simulink model "HvacMdlAirFlowDistbnFrnt"  (R2022b) (continued)
== system HvacMdlAirFlowDistbnFrnt == (continued)
  HvacAirMFlowEstimdForCtrlr_HvacAirMFlowEstimdForCtrlr/1 -> HvacMdlAirFlowDistbnFrnt_Runnable_sys/18(HvacAirMFlowEstimdForCtrlr_HvacAirMFlowEstimdForCtrlr_read)
  HvacCtrlrFanBoostReqLe_HvacCtrlrFanBoostReqLe/1 -> HvacMdlAirFlowDistbnFrnt_Runnable_sys/19(HvacCtrlrFanBoostReqLe_HvacCtrlrFanBoostReqLe_read)
  HvacCtrlrFanBoostReqRi_HvacCtrlrFanBoostReqRi/1 -> HvacMdlAirFlowDistbnFrnt_Runnable_sys/20(HvacCtrlrFanBoostReqRi_HvacCtrlrFanBoostReqRi_read)
  HvacCtrlrFanReqFrnt_HvacCtrlrFanReqFrnt/1 -> HvacMdlAirFlowDistbnFrnt_Runnable_sys/21(HvacCtrlrFanReqFrnt_HvacCtrlrFanReqFrnt_read)
  HvacCtrlrReqFrntForRowFirstForActrDefrst_HvacCtrlrReqFrntForRowFirstForActrDefrst/1 -> HvacMdlAirFlowDistbnFrnt_Runnable_sys/22(HvacCtrlrReqFrntForRowFirstForActrDefrst_HvacCtrlrReqFrntForRowFirstForActrDefrst_read)
  HvacCtrlrReqFrntForRowFirstForActrFlr_HvacCtrlrReqFrntForRowFirstForActrFlr/1 -> HvacMdlAirFlowDistbnFrnt_Runnable_sys/23(HvacCtrlrReqFrntForRowFirstForActrFlr_HvacCtrlrReqFrntForRowFirstForActrFlr_read)
  HvacCtrlrReqFrntForRowFirstForActrMod_HvacCtrlrReqFrntForRowFirstForActrMod/1 -> HvacMdlAirFlowDistbnFrnt_Runnable_sys/24(HvacCtrlrReqFrntForRowFirstForActrMod_HvacCtrlrReqFrntForRowFirstForActrMod_read)
  HvacCtrlrReqFrntForRowFirstForActrTLe_HvacCtrlrReqFrntForRowFirstForActrTLe/1 -> HvacMdlAirFlowDistbnFrnt_Runnable_sys/25(HvacCtrlrReqFrntForRowFirstForActrTLe_HvacCtrlrReqFrntForRowFirstForActrTLe_read)
  HvacCtrlrReqFrntForRowFirstForActrTRi_HvacCtrlrReqFrntForRowFirstForActrTRi/1 -> HvacMdlAirFlowDistbnFrnt_Runnable_sys/26(HvacCtrlrReqFrntForRowFirstForActrTRi_HvacCtrlrReqFrntForRowFirstForActrTRi_read)
  HvacCtrlrReqFrntForRowFirstForActrVent_HvacCtrlrReqFrntForRowFirstForActrVent/1 -> HvacMdlAirFlowDistbnFrnt_Runnable_sys/27(HvacCtrlrReqFrntForRowFirstForActrVent_HvacCtrlrReqFrntForRowFirstForActrVent_read)
  HvacCtrlrReqFrntForRowSecForActrFlr_HvacCtrlrReqFrntForRowSecForActrFlrLe/1 -> HvacMdlAirFlowDistbnFrnt_Runnable_sys/28(HvacCtrlrReqFrntForRowSecForActrFlr_HvacCtrlrReqFrntForRowSecForActrFlrLe_read)
  HvacCtrlrReqFrntForRowSecForActrTLe_HvacCtrlrReqFrntForRowSecForActrTLe/1 -> HvacMdlAirFlowDistbnFrnt_Runnable_sys/29(HvacCtrlrReqFrntForRowSecForActrTLe_HvacCtrlrReqFrntForRowSecForActrTLe_read)
  HvacCtrlrReqFrntForRowSecForActrTRi_HvacCtrlrReqFrntForRowSecForActrTRi/1 -> HvacMdlAirFlowDistbnFrnt_Runnable_sys/30(HvacCtrlrReqFrntForRowSecForActrTRi_HvacCtrlrReqFrntForRowSecForActrTRi_read)
  HvacCtrlrReqFrntForRowSecForActrVent_HvacCtrlrReqFrntForRowSecForActrVent/1 -> HvacMdlAirFlowDistbnFrnt_Runnable_sys/31(HvacCtrlrReqFrntForRowSecForActrVent_HvacCtrlrReqFrntForRowSecForActrVent_read)
  HvacFlowFrntReqForDefrst_HvacFlowFrntReqForDefrst/1 -> HvacMdlAirFlowDistbnFrnt_Runnable_sys/32(HvacFlowFrntReqForDefrst_HvacFlowFrntReqForDefrst_read)
  HvacFlowFrntReqForRowFirstAtFlr_HvacFlowFrntReqForRowFirstAtFlr/1 -> HvacMdlAirFlowDistbnFrnt_Runnable_sys/33(HvacFlowFrntReqForRowFirstAtFlr_HvacFlowFrntReqForRowFirstAtFlr_read)
  HvacFlowFrntReqForRowFirstAtFlr_HvacFlowFrntReqForRowFirstAtFlrLe/1 -> HvacMdlAirFlowDistbnFrnt_Runnable_sys/34(HvacFlowFrntReqForRowFirstAtFlr_HvacFlowFrntReqForRowFirstAtFlrLe_read)
  HvacFlowFrntReqForRowFirstAtFlr_HvacFlowFrntReqForRowFirstAtFlrRi/1 -> HvacMdlAirFlowDistbnFrnt_Runnable_sys/35(HvacFlowFrntReqForRowFirstAtFlr_HvacFlowFrntReqForRowFirstAtFlrRi_read)
  HvacFlowFrntReqForRowFirstAtVent_HvacFlowFrntReqForRowFirstAtVent/1 -> HvacMdlAirFlowDistbnFrnt_Runnable_sys/36(HvacFlowFrntReqForRowFirstAtVent_HvacFlowFrntReqForRowFirstAtVent_read)
  HvacFlowFrntReqForRowFirstAtVent_HvacFlowFrntReqForRowFirstAtVentLe/1 -> HvacMdlAirFlowDistbnFrnt_Runnable_sys/37(HvacFlowFrntReqForRowFirstAtVent_HvacFlowFrntReqForRowFirstAtVentLe_read)
  HvacFlowFrntReqForRowFirstAtVent_HvacFlowFrntReqForRowFirstAtVentLeMid/1 -> HvacMdlAirFlowDistbnFrnt_Runnable_sys/38(HvacFlowFrntReqForRowFirstAtVent_HvacFlowFrntReqForRowFirstAtVentLeMid_read)
  HvacFlowFrntReqForRowFirstAtVent_HvacFlowFrntReqForRowFirstAtVentLeSide/1 -> HvacMdlAirFlowDistbnFrnt_Runnable_sys/39(HvacFlowFrntReqForRowFirstAtVent_HvacFlowFrntReqForRowFirstAtVentLeSide_read)
  HvacFlowFrntReqForRowFirstAtVent_HvacFlowFrntReqForRowFirstAtVentRi/1 -> HvacMdlAirFlowDistbnFrnt_Runnable_sys/40(HvacFlowFrntReqForRowFirstAtVent_HvacFlowFrntReqForRowFirstAtVentRi_read)
  HvacFlowFrntReqForRowFirstAtVent_HvacFlowFrntReqForRowFirstAtVentRiMid/1 -> HvacMdlAirFlowDistbnFrnt_Runnable_sys/41(HvacFlowFrntReqForRowFirstAtVent_HvacFlowFrntReqForRowFirstAtVentRiMid_read)
  HvacFlowFrntReqForRowFirstAtVent_HvacFlowFrntReqForRowFirstAtVentRiSide/1 -> HvacMdlAirFlowDistbnFrnt_Runnable_sys/42(HvacFlowFrntReqForRowFirstAtVent_HvacFlowFrntReqForRowFirstAtVentRiSide_read)
  HvacFlowFrntReqForRowSecAtFlr_HvacFlowFrntReqForRowSecAtFlr/1 -> HvacMdlAirFlowDistbnFrnt_Runnable_sys/43(HvacFlowFrntReqForRowSecAtFlr_HvacFlowFrntReqForRowSecAtFlr_read)
  HvacFlowFrntReqForRowSecAtFlr_HvacFlowFrntReqForRowSecAtFlrLe/1 -> HvacMdlAirFlowDistbnFrnt_Runnable_sys/44(HvacFlowFrntReqForRowSecAtFlr_HvacFlowFrntReqForRowSecAtFlrLe_read)
  HvacFlowFrntReqForRowSecAtFlr_HvacFlowFrntReqForRowSecAtFlrRi/1 -> HvacMdlAirFlowDistbnFrnt_Runnable_sys/45(HvacFlowFrntReqForRowSecAtFlr_HvacFlowFrntReqForRowSecAtFlrRi_read)
  HvacFlowFrntReqForRowSecAtVent_HvacFlowFrntReqForRowSecAtVent/1 -> HvacMdlAirFlowDistbnFrnt_Runnable_sys/46(HvacFlowFrntReqForRowSecAtVent_HvacFlowFrntReqForRowSecAtVent_read)
  HvacFlowFrntReqForRowSecAtVent_HvacFlowFrntReqForRowSecAtVentLe/1 -> HvacMdlAirFlowDistbnFrnt_Runnable_sys/47(HvacFlowFrntReqForRowSecAtVent_HvacFlowFrntReqForRowSecAtVentLe_read)
  HvacFlowFrntReqForRowSecAtVent_HvacFlowFrntReqForRowSecAtVentRi/1 -> HvacMdlAirFlowDistbnFrnt_Runnable_sys/48(HvacFlowFrntReqForRowSecAtVent_HvacFlowFrntReqForRowSecAtVentRi_read)
  NotAllwdForMaxReqReqdForRowSecAtVent_NotAllwdForMaxReqReqdForRowSecAtVent/1 -> HvacMdlAirFlowDistbnFrnt_Runnable_sys/49(NotAllwdForMaxReqReqdForRowSecAtVent_NotAllwdForMaxReqReqdForRowSecAtVent_read)
  OvrdForMaxReqForActrForDefrst_OvrdForMaxReqForActrForDefrst/1 -> HvacMdlAirFlowDistbnFrnt_Runnable_sys/50(OvrdForMaxReqForActrForDefrst_OvrdForMaxReqForActrForDefrst_read)
  OvrdForMaxReqForActrForRowFirstAtFlr_OvrdForMaxReqForActrForRowFirstAtFlr/1 -> HvacMdlAirFlowDistbnFrnt_Runnable_sys/51(OvrdForMaxReqForActrForRowFirstAtFlr_OvrdForMaxReqForActrForRowFirstAtFlr_read)
  OvrdForMaxReqForActrForRowFirstAtFlr_OvrdForMaxReqForActrForRowFirstAtFlrLe/1 -> HvacMdlAirFlowDistbnFrnt_Runnable_sys/52(OvrdForMaxReqForActrForRowFirstAtFlr_OvrdForMaxReqForActrForRowFirstAtFlrLe_read)
  OvrdForMaxReqForActrForRowFirstAtFlr_OvrdForMaxReqForActrForRowFirstAtFlrRi/1 -> HvacMdlAirFlowDistbnFrnt_Runnable_sys/53(OvrdForMaxReqForActrForRowFirstAtFlr_OvrdForMaxReqForActrForRowFirstAtFlrRi_read)
  OvrdForMaxReqForActrForRowFirstAtVent_OvrdForMaxReqForActrForRowFirstAtVent/1 -> HvacMdlAirFlowDistbnFrnt_Runnable_sys/54(OvrdForMaxReqForActrForRowFirstAtVent_OvrdForMaxReqForActrForRowFirstAtVent_read)
  OvrdForMaxReqForActrForRowFirstAtVent_OvrdForMaxReqForActrForRowFirstAtVentLe/1 -> HvacMdlAirFlowDistbnFrnt_Runnable_sys/55(OvrdForMaxReqForActrForRowFirstAtVent_OvrdForMaxReqForActrForRowFirstAtVentLe_read)
  OvrdForMaxReqForActrForRowFirstAtVent_OvrdForMaxReqForActrForRowFirstAtVentRi/1 -> HvacMdlAirFlowDistbnFrnt_Runnable_sys/56(OvrdForMaxReqForActrForRowFirstAtVent_OvrdForMaxReqForActrForRowFirstAtVentRi_read)
  OvrdForMaxReqForActrForRowFirstAtVent_OvrdForMaxReqForActrForRowFirstAtVentSideLe/1 -> HvacMdlAirFlowDistbnFrnt_Runnable_sys/57(OvrdForMaxReqForActrForRowFirstAtVent_OvrdForMaxReqForActrForRowFirstAtVentSideLe_read)
  OvrdForMaxReqForActrForRowFirstAtVent_OvrdForMaxReqForActrForRowFirstAtVentSideRi/1 -> HvacMdlAirFlowDistbnFrnt_Runnable_sys/58(OvrdForMaxReqForActrForRowFirstAtVent_OvrdForMaxReqForActrForRowFirstAtVentSideRi_read)
  OvrdForMaxReqForActrForRowSecAtFlr_OvrdForMaxReqForActrForRowSecAtFlr/1 -> HvacMdlAirFlowDistbnFrnt_Runnable_sys/59(OvrdForMaxReqForActrForRowSecAtFlr_OvrdForMaxReqForActrForRowSecAtFlr_read)
  OvrdForMaxReqForActrForRowSecAtFlr_OvrdForMaxReqForActrForRowSecAtFlrLe/1 -> HvacMdlAirFlowDistbnFrnt_Runnable_sys/60(OvrdForMaxReqForActrForRowSecAtFlr_OvrdForMaxReqForActrForRowSecAtFlrLe_read)
  OvrdForMaxReqForActrForRowSecAtFlr_OvrdForMaxReqForActrForRowSecAtFlrRi/1 -> HvacMdlAirFlowDistbnFrnt_Runnable_sys/61(OvrdForMaxReqForActrForRowSecAtFlr_OvrdForMaxReqForActrForRowSecAtFlrRi_read)
  OvrdForMaxReqForActrForRowSecAtVent_OvrdForMaxReqForActrForRowSecAtVent/1 -> HvacMdlAirFlowDistbnFrnt_Runnable_sys/62(OvrdForMaxReqForActrForRowSecAtVent_OvrdForMaxReqForActrForRowSecAtVent_read)
  OvrdForMaxReqForActrForRowSecAtVent_OvrdForMaxReqForActrForRowSecAtVentLe/1 -> HvacMdlAirFlowDistbnFrnt_Runnable_sys/63(OvrdForMaxReqForActrForRowSecAtVent_OvrdForMaxReqForActrForRowSecAtVentLe_read)
  OvrdForMaxReqForActrForRowSecAtVent_OvrdForMaxReqForActrForRowSecAtVentRi/1 -> HvacMdlAirFlowDistbnFrnt_Runnable_sys/64(OvrdForMaxReqForActrForRowSecAtVent_OvrdForMaxReqForActrForRowSecAtVentRi_read)
  ClimaCfgForNrOfTSetg1_ClimaCfgForNrOfTSetg1/1 -> HvacMdlAirFlowDistbnFrnt_Runnable_sys/65(ClimaCfgForNrOfTSetg1_ClimaCfgForNrOfTSetg1_read)
  HvacCtrlrReqFrntForRowFirstForActrFlr_HvacCtrlrReqFrntForRowFirstForActrFlrLe/1 -> HvacMdlAirFlowDistbnFrnt_Runnable_sys/66(HvacCtrlrReqFrntForRowFirstForActrFlr_HvacCtrlrReqFrntForRowFirstForActrFlrLe_read)
  HvacCtrlrReqFrntForRowFirstForActrFlr_HvacCtrlrReqFrntForRowFirstForActrFlrRi/1 -> HvacMdlAirFlowDistbnFrnt_Runnable_sys/67(HvacCtrlrReqFrntForRowFirstForActrFlr_HvacCtrlrReqFrntForRowFirstForActrFlrRi_read)
  HvacCtrlrReqFrntForRowFirstForActrVent_HvacCtrlrReqFrntForRowFirstForActrVentLe/1 -> HvacMdlAirFlowDistbnFrnt_Runnable_sys/68(HvacCtrlrReqFrntForRowFirstForActrVent_HvacCtrlrReqFrntForRowFirstForActrVentLe_read)
  HvacCtrlrReqFrntForRowFirstForActrVent_HvacCtrlrReqFrntForRowFirstForActrVentRi/1 -> HvacMdlAirFlowDistbnFrnt_Runnable_sys/69(HvacCtrlrReqFrntForRowFirstForActrVent_HvacCtrlrReqFrntForRowFirstForActrVentRi_read)
  HvacCtrlrReqFrntForRowFirstForActrVent_HvacCtrlrReqFrntForRowFirstForActrVentSideLe/1 -> HvacMdlAirFlowDistbnFrnt_Runnable_sys/70(HvacCtrlrReqFrntForRowFirstForActrVent_HvacCtrlrReqFrntForRowFirstForActrVentSideLe_read)
  HvacCtrlrReqFrntForRowFirstForActrVent_HvacCtrlrReqFrntForRowFirstForActrVentSideRi/1 -> HvacMdlAirFlowDistbnFrnt_Runnable_sys/71(HvacCtrlrReqFrntForRowFirstForActrVent_HvacCtrlrReqFrntForRowFirstForActrVentSideRi_read)
  HvacCtrlrReqFrntForRowFirstLeForActrMod_HvacCtrlrReqFrntForRowFirstLeForActrMod/1 -> HvacMdlAirFlowDistbnFrnt_Runnable_sys/72(HvacCtrlrReqFrntForRowFirstLeForActrMod_HvacCtrlrReqFrntForRowFirstLeForActrMod_read)
  HvacCtrlrReqFrntForRowSecForActrFlr_HvacCtrlrReqFrntForRowSecForActrFlr/1 -> HvacMdlAirFlowDistbnFrnt_Runnable_sys/73(HvacCtrlrReqFrntForRowSecForActrFlr_HvacCtrlrReqFrntForRowSecForActrFlr_read)
  HvacCtrlrReqFrntForRowSecForActrFlr_HvacCtrlrReqFrntForRowSecForActrFlrRi/1 -> HvacMdlAirFlowDistbnFrnt_Runnable_sys/74(HvacCtrlrReqFrntForRowSecForActrFlr_HvacCtrlrReqFrntForRowSecForActrFlrRi_read)
  HvacCtrlrReqFrntForRowSecForActrModLe_HvacCtrlrReqFrntForRowSecForActrModLe/1 -> HvacMdlAirFlowDistbnFrnt_Runnable_sys/75(HvacCtrlrReqFrntForRowSecForActrModLe_HvacCtrlrReqFrntForRowSecForActrModLe_read)
  HvacCtrlrReqFrntForRowSecForActrModRi_HvacCtrlrReqFrntForRowSecForActrModRi/1 -> HvacMdlAirFlowDistbnFrnt_Runnable_sys/76(HvacCtrlrReqFrntForRowSecForActrModRi_HvacCtrlrReqFrntForRowSecForActrModRi_read)
  ExtVentToastFrmClima_Prkg_ExtVentToastFrmClima/1 -> HvacMdlAirFlowDistbnFrnt_Runnable_sys/77(ExtVentToastFrmClima_Prkg_ExtVentToastFrmClima_read)
  ActrPosnFrntActForRowFirstModDistbnLe_ActrPosnFrntActForRowFirstModDistbnLe/1 -> HvacMdlAirFlowDistbnFrnt_Runnable_sys/78(ActrPosnFrntActForRowFirstModDistbnLe_ActrPosnFrntActForRowFirstModDistbnLe_read)
  ActrPosnFrntActForRowSecModDistbnLe_ActrPosnFrntActForRowSecModDistbnLe/1 -> HvacMdlAirFlowDistbnFrnt_Runnable_sys/79(ActrPosnFrntActForRowSecModDistbnLe_ActrPosnFrntActForRowSecModDistbnLe_read)
  ActrPosnFrntActForRowSecModDistbnRi_ActrPosnFrntActForRowSecModDistbnRi/1 -> HvacMdlAirFlowDistbnFrnt_Runnable_sys/80(ActrPosnFrntActForRowSecModDistbnRi_ActrPosnFrntActForRowSecModDistbnRi_read)
  AirFlapPosnFrntActForRowFirstForActrDefrst_AirFlapPosnFrntActForRowFirstForActrDefrst/1 -> HvacMdlAirFlowDistbnFrnt_Runnable_sys/81(AirFlapPosnFrntActForRowFirstForActrDefrst_AirFlapPosnFrntActForRowFirstForActrDefrst_read)
  ClimaAtRowSecActvNgcc_ClimaAtRowSecActv/1 -> HvacMdlAirFlowDistbnFrnt_Runnable_sys/82(ClimaAtRowSecActvNgcc_ClimaAtRowSecActv_read)
  ClimaAtRowSecActvNgcc_ClimaAtRowSecActvLe/1 -> HvacMdlAirFlowDistbnFrnt_Runnable_sys/83(ClimaAtRowSecActvNgcc_ClimaAtRowSecActvLe_read)
  ClimaAtRowSecActvNgcc_ClimaAtRowSecActvRi/1 -> HvacMdlAirFlowDistbnFrnt_Runnable_sys/84(ClimaAtRowSecActvNgcc_ClimaAtRowSecActvRi_read)
  ClimaCfgForHvacRePrsnt1_ClimaCfgForHvacRePrsnt1/1 -> HvacMdlAirFlowDistbnFrnt_Runnable_sys/85(ClimaCfgForHvacRePrsnt1_ClimaCfgForHvacRePrsnt1_read)
  ClimaCfgForPTCInFrontHVAC_ClimaCfgForPTCInFrontHVAC/1 -> HvacMdlAirFlowDistbnFrnt_Runnable_sys/86(ClimaCfgForPTCInFrontHVAC_ClimaCfgForPTCInFrontHVAC_read)
  ClimaCfgHvacForFrntLeTempActr_ClimaCfgHvacForFrntLeTempActr/1 -> HvacMdlAirFlowDistbnFrnt_Runnable_sys/87(ClimaCfgHvacForFrntLeTempActr_ClimaCfgHvacForFrntLeTempActr_read)
  ClimaCfgHvacForFrntRiTempActr_ClimaCfgHvacForFrntRiTempActr/1 -> HvacMdlAirFlowDistbnFrnt_Runnable_sys/88(ClimaCfgHvacForFrntRiTempActr_ClimaCfgHvacForFrntRiTempActr_read)
  ClimaCfgReqVersionReplace_ClimaCfgReqVersionReplace/1 -> HvacMdlAirFlowDistbnFrnt_Runnable_sys/89(ClimaCfgReqVersionReplace_ClimaCfgReqVersionReplace_read)
  HvacTempFlapActlPosnFrstRowLe_HvacTempFlapActlPosnFrstRowLe/1 -> HvacMdlAirFlowDistbnFrnt_Runnable_sys/90(HvacTempFlapActlPosnFrstRowLe_HvacTempFlapActlPosnFrstRowLe_read)
  HvacTempFlapActlPosnFrstRowRi_HvacTempFlapActlPosnFrstRowRi/1 -> HvacMdlAirFlowDistbnFrnt_Runnable_sys/91(HvacTempFlapActlPosnFrstRowRi_HvacTempFlapActlPosnFrstRowRi_read)
  HvacTempFlapActlPosnSecRowLe_HvacTempFlapActlPosnSecRowLe/1 -> HvacMdlAirFlowDistbnFrnt_Runnable_sys/92(HvacTempFlapActlPosnSecRowLe_HvacTempFlapActlPosnSecRowLe_read)
  HvacTempFlapActlPosnSecRowRi_HvacTempFlapActlPosnSecRowRi/1 -> HvacMdlAirFlowDistbnFrnt_Runnable_sys/93(HvacTempFlapActlPosnSecRowRi_HvacTempFlapActlPosnSecRowRi_read)
  ManModeForRowFirstLeForModelDial_ManModeForRowFirstLeForModelDial/1 -> HvacMdlAirFlowDistbnFrnt_Runnable_sys/94(ManModeForRowFirstLeForModelDial_ManModeForRowFirstLeForModelDial_read)
  ManModeForRowFirstRiForModelDial_ManModeForRowFirstRiForModelDial/1 -> HvacMdlAirFlowDistbnFrnt_Runnable_sys/95(ManModeForRowFirstRiForModelDial_ManModeForRowFirstRiForModelDial_read)
  ManModeForRowSecLeForModelDial_ManModeForRowSecLeForModelDial/1 -> HvacMdlAirFlowDistbnFrnt_Runnable_sys/96(ManModeForRowSecLeForModelDial_ManModeForRowSecLeForModelDial_read)
  ManModeForRowSecRiForModelDial_ManModeForRowSecRiForModelDial/1 -> HvacMdlAirFlowDistbnFrnt_Runnable_sys/97(ManModeForRowSecRiForModelDial_ManModeForRowSecRiForModelDial_read)
  HvacMdlAirFlowDistbnFrnt_Runnable_sys/1(HvacHeatElmAirMFlowEstimdFirstLeNgcc_HvacHeatElmAirMFlowEstimdFirstLeNgcc_write) -> HvacHeatElmAirMFlowEstimdFirstLeNgcc_HvacHeatElmAirMFlowEstimdFirstLeNgcc/1
  HvacMdlAirFlowDistbnFrnt_Runnable_sys/2(HvacHeatElmAirMFlowEstimdFirstRiNgcc_HvacHeatElmAirMFlowEstimdFirstRiNgcc_write) -> HvacHeatElmAirMFlowEstimdFirstRiNgcc_HvacHeatElmAirMFlowEstimdFirstRiNgcc/1
  HvacMdlAirFlowDistbnFrnt_Runnable_sys/3(HvacHeatElmAirMFlowEstimdSecLeNgcc_HvacHeatElmAirMFlowEstimdSecLeNgcc_write) -> HvacHeatElmAirMFlowEstimdSecLeNgcc_HvacHeatElmAirMFlowEstimdSecLeNgcc/1
  HvacMdlAirFlowDistbnFrnt_Runnable_sys/4(FacOfSecVent_FacOfSecVent_write) -> FacOfSecVent_FacOfSecVent/1
  HvacMdlAirFlowDistbnFrnt_Runnable_sys/5(FacOfVentLeFlow_FacOfVentLeFlow_write) -> FacOfVentLeFlow_FacOfVentLeFlow/1
  HvacMdlAirFlowDistbnFrnt_Runnable_sys/6(FacOfVentRiFlow_FacOfVentRiFlow_write) -> FacOfVentRiFlow_FacOfVentRiFlow/1
  HvacMdlAirFlowDistbnFrnt_Runnable_sys/7(FacOfVentSideLeFlow_FacOfVentSideLeFlow_write) -> FacOfVentSideLeFlow_FacOfVentSideLeFlow/1
  HvacMdlAirFlowDistbnFrnt_Runnable_sys/8(FacOfVentSideRiFlow_FacOfVentSideRiFlow_write) -> FacOfVentSideRiFlow_FacOfVentSideRiFlow/1
  HvacMdlAirFlowDistbnFrnt_Runnable_sys/9(HvacAirFlowAddlForFanBoost_HvacAirFlowAddlForFanBoost_write) -> HvacAirFlowAddlForFanBoost_HvacAirFlowAddlForFanBoost/1
  HvacMdlAirFlowDistbnFrnt_Runnable_sys/10(HvacAirMFlowEstimdInHeatElm_HvacAirMFlowEstimdInHeatElm_write) -> HvacAirMFlowEstimdInHeatElm_HvacAirMFlowEstimdInHeatElm/1
  HvacMdlAirFlowDistbnFrnt_Runnable_sys/11(HvacFlowFrntActForDefrst_HvacFlowFrntActForDefrst_write) -> HvacFlowFrntActForDefrst_HvacFlowFrntActForDefrst/1
  HvacMdlAirFlowDistbnFrnt_Runnable_sys/12(HvacFlowFrntActForRowFirstAtFlr_HvacFlowFrntActForRowFirstAtFlr_write) -> HvacFlowFrntActForRowFirstAtFlr_HvacFlowFrntActForRowFirstAtFlr/1
  HvacMdlAirFlowDistbnFrnt_Runnable_sys/13(HvacFlowFrntActForRowFirstAtVent_HvacFlowFrntActForRowFirstAtVent_write) -> HvacFlowFrntActForRowFirstAtVent_HvacFlowFrntActForRowFirstAtVent/1
  HvacMdlAirFlowDistbnFrnt_Runnable_sys/14(HvacFlowFrntActForRowSecAtFlr_HvacFlowFrntActForRowSecAtFlr_write) -> HvacFlowFrntActForRowSecAtFlr_HvacFlowFrntActForRowSecAtFlr/1
  HvacMdlAirFlowDistbnFrnt_Runnable_sys/15(HvacFlowFrntActForRowSecAtVent_HvacFlowFrntActForRowSecAtVent_write) -> HvacFlowFrntActForRowSecAtVent_HvacFlowFrntActForRowSecAtVent/1
  HvacMdlAirFlowDistbnFrnt_Runnable_sys/16(HvacFlowFrntActForRowSecAtVent_HvacFlowFrntActForRowSecAtVentLe_write) -> HvacFlowFrntActForRowSecAtVent_HvacFlowFrntActForRowSecAtVentLe/1
  HvacMdlAirFlowDistbnFrnt_Runnable_sys/17(HvacFlowFrntActForRowSecAtVent_HvacFlowFrntActForRowSecAtVentRi_write) -> HvacFlowFrntActForRowSecAtVent_HvacFlowFrntActForRowSecAtVentRi/1
  HvacMdlAirFlowDistbnFrnt_Runnable_sys/18(HvacFlowFrntActForRowThrdAtFlr_HvacFlowFrntActForRowThrdAtFlr_write) -> HvacFlowFrntActForRowThrdAtFlr_HvacFlowFrntActForRowThrdAtFlr/1
  HvacMdlAirFlowDistbnFrnt_Runnable_sys/19(HvacFlowFrntForRowFirstAtDefrstLe_HvacFlowFrntForRowFirstAtDefrstLe_write) -> HvacFlowFrntForRowFirstAtDefrstLe_HvacFlowFrntForRowFirstAtDefrstLe/1
  HvacMdlAirFlowDistbnFrnt_Runnable_sys/20(HvacFlowFrntForRowFirstAtDefrstRi_HvacFlowFrntForRowFirstAtDefrstRi_write) -> HvacFlowFrntForRowFirstAtDefrstRi_HvacFlowFrntForRowFirstAtDefrstRi/1
  HvacMdlAirFlowDistbnFrnt_Runnable_sys/21(HvacFlowFrntForRowFirstAtDefrstSideLe_HvacFlowFrntForRowFirstAtDefrstSideLe_write) -> HvacFlowFrntForRowFirstAtDefrstSideLe_HvacFlowFrntForRowFirstAtDefrstSideLe/1
  HvacMdlAirFlowDistbnFrnt_Runnable_sys/22(HvacFlowFrntForRowFirstAtDefrstSideRi_HvacFlowFrntForRowFirstAtDefrstSideRi_write) -> HvacFlowFrntForRowFirstAtDefrstSideRi_HvacFlowFrntForRowFirstAtDefrstSideRi/1
  HvacMdlAirFlowDistbnFrnt_Runnable_sys/23(HvacFlowFrntForRowFirstAtFlrLe_HvacFlowFrntForRowFirstAtFlrLe_write) -> HvacFlowFrntForRowFirstAtFlrLe_HvacFlowFrntForRowFirstAtFlrLe/1
  HvacMdlAirFlowDistbnFrnt_Runnable_sys/24(HvacFlowFrntForRowFirstAtFlrRi_HvacFlowFrntForRowFirstAtFlrRi_write) -> HvacFlowFrntForRowFirstAtFlrRi_HvacFlowFrntForRowFirstAtFlrRi/1
  HvacMdlAirFlowDistbnFrnt_Runnable_sys/25(HvacFlowFrntForRowFirstAtVentLe_HvacFlowFrntForRowFirstAtVentLe_write) -> HvacFlowFrntForRowFirstAtVentLe_HvacFlowFrntForRowFirstAtVentLe/1
  HvacMdlAirFlowDistbnFrnt_Runnable_sys/26(HvacFlowFrntForRowFirstAtVentRi_HvacFlowFrntForRowFirstAtVentRi_write) -> HvacFlowFrntForRowFirstAtVentRi_HvacFlowFrntForRowFirstAtVentRi/1
  HvacMdlAirFlowDistbnFrnt_Runnable_sys/27(HvacFlowFrntForRowFirstAtVentSideLe_HvacFlowFrntForRowFirstAtVentSideLe_write) -> HvacFlowFrntForRowFirstAtVentSideLe_HvacFlowFrntForRowFirstAtVentSideLe/1
  HvacMdlAirFlowDistbnFrnt_Runnable_sys/28(HvacFlowFrntForRowFirstAtVentSideRi_HvacFlowFrntForRowFirstAtVentSideRi_write) -> HvacFlowFrntForRowFirstAtVentSideRi_HvacFlowFrntForRowFirstAtVentSideRi/1
  HvacMdlAirFlowDistbnFrnt_Runnable_sys/29(HvacFlowFrntForRowSecAtFlrLe_HvacFlowFrntForRowSecAtFlrLe_write) -> HvacFlowFrntForRowSecAtFlrLe_HvacFlowFrntForRowSecAtFlrLe/1
  HvacMdlAirFlowDistbnFrnt_Runnable_sys/30(HvacFlowFrntForRowSecAtFlrRi_HvacFlowFrntForRowSecAtFlrRi_write) -> HvacFlowFrntForRowSecAtFlrRi_HvacFlowFrntForRowSecAtFlrRi/1
  HvacMdlAirFlowDistbnFrnt_Runnable_sys/31(HvacFlowFrntForRowSecAtVentLe_HvacFlowFrntForRowSecAtVentLe_write) -> HvacFlowFrntForRowSecAtVentLe_HvacFlowFrntForRowSecAtVentLe/1
  HvacMdlAirFlowDistbnFrnt_Runnable_sys/32(HvacFlowFrntForRowSecAtVentRi_HvacFlowFrntForRowSecAtVentRi_write) -> HvacFlowFrntForRowSecAtVentRi_HvacFlowFrntForRowSecAtVentRi/1
  HvacMdlAirFlowDistbnFrnt_Runnable_sys/33(HvacFlowFrntForRowSecAtVentSideLe_HvacFlowFrntForRowSecAtVentSideLe_write) -> HvacFlowFrntForRowSecAtVentSideLe_HvacFlowFrntForRowSecAtVentSideLe/1
  HvacMdlAirFlowDistbnFrnt_Runnable_sys/34(HvacFlowFrntForRowSecAtVentSideRi_HvacFlowFrntForRowSecAtVentSideRi_write) -> HvacFlowFrntForRowSecAtVentSideRi_HvacFlowFrntForRowSecAtVentSideRi/1
  HvacMdlAirFlowDistbnFrnt_Runnable_sys/35(HvacFlowFrntForRowThrdAtFlrLe_HvacFlowFrntForRowThrdAtFlrLe_write) -> HvacFlowFrntForRowThrdAtFlrLe_HvacFlowFrntForRowThrdAtFlrLe/1
  HvacMdlAirFlowDistbnFrnt_Runnable_sys/36(HvacFlowFrntForRowThrdAtFlrRi_HvacFlowFrntForRowThrdAtFlrRi_write) -> HvacFlowFrntForRowThrdAtFlrRi_HvacFlowFrntForRowThrdAtFlrRi/1
  HvacMdlAirFlowDistbnFrnt_Runnable_sys/37(HvacFlrsFrnt_HvacFlrsFrnt_write) -> HvacFlrsFrnt_HvacFlrsFrnt/1
  HvacMdlAirFlowDistbnFrnt_Runnable_sys/38(MaxReqReqdFrntForDefrst_MaxReqReqdFrntForDefrst_write) -> MaxReqReqdFrntForDefrst_MaxReqReqdFrntForDefrst/1
  HvacMdlAirFlowDistbnFrnt_Runnable_sys/39(MaxReqReqdFrntForRowFirstAtFlr_MaxReqReqdFrntForRowFirstAtFlr_write) -> MaxReqReqdFrntForRowFirstAtFlr_MaxReqReqdFrntForRowFirstAtFlr/1
  HvacMdlAirFlowDistbnFrnt_Runnable_sys/40(MaxReqReqdFrntForRowFirstAtFlr_MaxReqReqdFrntForRowFirstAtFlrLe_write) -> MaxReqReqdFrntForRowFirstAtFlr_MaxReqReqdFrntForRowFirstAtFlrLe/1
  HvacMdlAirFlowDistbnFrnt_Runnable_sys/41(MaxReqReqdFrntForRowFirstAtFlr_MaxReqReqdFrntForRowFirstAtFlrRi_write) -> MaxReqReqdFrntForRowFirstAtFlr_MaxReqReqdFrntForRowFirstAtFlrRi/1
  HvacMdlAirFlowDistbnFrnt_Runnable_sys/42(MaxReqReqdFrntForRowFirstAtVent_MaxReqReqdFrntForRowFirstAtVent_write) -> MaxReqReqdFrntForRowFirstAtVent_MaxReqReqdFrntForRowFirstAtVent/1
  HvacMdlAirFlowDistbnFrnt_Runnable_sys/43(MaxReqReqdFrntForRowSecAtFlr_MaxReqReqdFrntForRowSecAtFlr_write) -> MaxReqReqdFrntForRowSecAtFlr_MaxReqReqdFrntForRowSecAtFlr/1
  HvacMdlAirFlowDistbnFrnt_Runnable_sys/44(MaxReqReqdFrntForRowSecAtVent_MaxReqReqdFrntForRowSecAtVent_write) -> MaxReqReqdFrntForRowSecAtVent_MaxReqReqdFrntForRowSecAtVent/1
  HvacMdlAirFlowDistbnFrnt_Runnable_sys/45(VCCMaxReqReqdForActrFrntEnumReserved_VCCMaxReqReqdForActrFrntEnumReserved_write) -> VCCMaxReqReqdForActrFrntEnumReserved_VCCMaxReqReqdForActrFrntEnumReserved/1
  HvacMdlAirFlowDistbnFrnt_Runnable_sys/46(HvacFlowFrntActForRowFirstAtFlr_HvacFlowFrntActForRowFirstAtFlrLe_write) -> HvacFlowFrntActForRowFirstAtFlr_HvacFlowFrntActForRowFirstAtFlrLe/1
  HvacMdlAirFlowDistbnFrnt_Runnable_sys/47(HvacFlowFrntActForRowFirstAtFlr_HvacFlowFrntActForRowFirstAtFlrRi_write) -> HvacFlowFrntActForRowFirstAtFlr_HvacFlowFrntActForRowFirstAtFlrRi/1
  HvacMdlAirFlowDistbnFrnt_Runnable_sys/48(HvacFlowFrntActForRowFirstAtVent_HvacFlowFrntActForRowFirstAtVentLe_write) -> HvacFlowFrntActForRowFirstAtVent_HvacFlowFrntActForRowFirstAtVentLe/1
  HvacMdlAirFlowDistbnFrnt_Runnable_sys/49(HvacFlowFrntActForRowFirstAtVent_HvacFlowFrntActForRowFirstAtVentRi_write) -> HvacFlowFrntActForRowFirstAtVent_HvacFlowFrntActForRowFirstAtVentRi/1
  HvacMdlAirFlowDistbnFrnt_Runnable_sys/50(HvacFlowFrntActForRowFirstAtVent_HvacFlowFrntActForRowFirstAtVentSideLe_write) -> HvacFlowFrntActForRowFirstAtVent_HvacFlowFrntActForRowFirstAtVentSideLe/1
  HvacMdlAirFlowDistbnFrnt_Runnable_sys/51(HvacFlowFrntActForRowFirstAtVent_HvacFlowFrntActForRowFirstAtVentSideRi_write) -> HvacFlowFrntActForRowFirstAtVent_HvacFlowFrntActForRowFirstAtVentSideRi/1
  HvacMdlAirFlowDistbnFrnt_Runnable_sys/52(HvacFlowFrntActForRowSecAtFlr_HvacFlowFrntActForRowSecAtFlrLe_write) -> HvacFlowFrntActForRowSecAtFlr_HvacFlowFrntActForRowSecAtFlrLe/1
  HvacMdlAirFlowDistbnFrnt_Runnable_sys/53(HvacFlowFrntActForRowSecAtFlr_HvacFlowFrntActForRowSecAtFlrRi_write) -> HvacFlowFrntActForRowSecAtFlr_HvacFlowFrntActForRowSecAtFlrRi/1
  MaxReqReqdFrntForRowFirstAtVent_MaxReqReqdFrntForRowFirstAtVentLe_merge/1 -> MaxReqReqdFrntForRowFirstAtVent_MaxReqReqdFrntForRowFirstAtVentLe/1
  MaxReqReqdFrntForRowFirstAtVent_MaxReqReqdFrntForRowFirstAtVentRi_merge/1 -> MaxReqReqdFrntForRowFirstAtVent_MaxReqReqdFrntForRowFirstAtVentRi/1
  HvacMdlAirFlowDistbnFrnt_Runnable_sys/56(MaxReqReqdFrntForRowFirstAtVent_MaxReqReqdFrntForRowFirstAtVentSideLe_write) -> MaxReqReqdFrntForRowFirstAtVent_MaxReqReqdFrntForRowFirstAtVentSideLe/1
  HvacMdlAirFlowDistbnFrnt_Runnable_sys/57(MaxReqReqdFrntForRowFirstAtVent_MaxReqReqdFrntForRowFirstAtVentSideRi_write) -> MaxReqReqdFrntForRowFirstAtVent_MaxReqReqdFrntForRowFirstAtVentSideRi/1
  HvacMdlAirFlowDistbnFrnt_Runnable_sys/58(MaxReqReqdFrntForRowSecAtFlr_MaxReqReqdFrntForRowSecAtFlrLe_write) -> MaxReqReqdFrntForRowSecAtFlr_MaxReqReqdFrntForRowSecAtFlrLe/1
  HvacMdlAirFlowDistbnFrnt_Runnable_sys/59(MaxReqReqdFrntForRowSecAtFlr_MaxReqReqdFrntForRowSecAtFlrRi_write) -> MaxReqReqdFrntForRowSecAtFlr_MaxReqReqdFrntForRowSecAtFlrRi/1
  HvacMdlAirFlowDistbnFrnt_Runnable_sys/60(MaxReqReqdFrntForRowSecAtVent_MaxReqReqdFrntForRowSecAtVentLe_write) -> MaxReqReqdFrntForRowSecAtVent_MaxReqReqdFrntForRowSecAtVentLe/1
  HvacMdlAirFlowDistbnFrnt_Runnable_sys/61(MaxReqReqdFrntForRowSecAtVent_MaxReqReqdFrntForRowSecAtVentRi_write) -> MaxReqReqdFrntForRowSecAtVent_MaxReqReqdFrntForRowSecAtVentRi/1
  HvacMdlAirFlowDistbnFrnt_Runnable_sys/55(MaxReqReqdFrntForRowFirstAtVent_MaxReqReqdFrntForRowFirstAtVentRi_write) -> [MaxReqReqdFrntForRowFirstAtVent_MaxReqReqdFrnt_a0f2095ec0cb5985]
  [MaxReqReqdFrntForRowFirstAtVent_MaxReqReqdFrnt_a0f2095ec0cb5985] -> MaxReqReqdFrntForRowFirstAtVent_MaxReqReqdFrntForRowFirstAtVentRi_merge/2
  HvacMdlAirFlowDistbnFrnt_Init/2(MaxReqReqdFrntForRowFirstAtVent_MaxReqReqdFrntForRowFirstAtVentRi_write) -> [MaxReqReqdFrntForRowFirstAtVent_MaxReqReqdFrnt_1a730eb18aa1a969]
  [MaxReqReqdFrntForRowFirstAtVent_MaxReqReqdFrnt_1a730eb18aa1a969] -> MaxReqReqdFrntForRowFirstAtVent_MaxReqReqdFrntForRowFirstAtVentRi_merge/1
  HvacMdlAirFlowDistbnFrnt_Runnable_sys/54(MaxReqReqdFrntForRowFirstAtVent_MaxReqReqdFrntForRowFirstAtVentLe_write) -> [MaxReqReqdFrntForRowFirstAtVent_MaxReqReqdFrnt_795653b2da2ba8ea]
  [MaxReqReqdFrntForRowFirstAtVent_MaxReqReqdFrnt_795653b2da2ba8ea] -> MaxReqReqdFrntForRowFirstAtVent_MaxReqReqdFrntForRowFirstAtVentLe_merge/2
  HvacMdlAirFlowDistbnFrnt_Init/1(MaxReqReqdFrntForRowFirstAtVent_MaxReqReqdFrntForRowFirstAtVentLe_write) -> [MaxReqReqdFrntForRowFirstAtVent_MaxReqReqdFrnt_ca95337f94ce6a9c]
  [MaxReqReqdFrntForRowFirstAtVent_MaxReqReqdFrnt_ca95337f94ce6a9c] -> MaxReqReqdFrntForRowFirstAtVent_MaxReqReqdFrntForRowFirstAtVentLe_merge/1
  HvacMdlAirFlowDistbnFrnt_Runnable/1 -> HvacMdlAirFlowDistbnFrnt_Runnable_sys/Trigger
